simulink model "hyd_hyb_2_int_cont_1_new3_5cyc"  (Simulink 7.7)
settings: Solver=ode4  StartTime=0  StopTime=600  FixedStep=0.01  MaxStep=.4  RelTol=1e-8
InitFcn:
  | const_3_new
values from neighbouring files (symbols this model uses):
  Af = 6.3    [brk_enrg.m]    (also in brk_enrg_1.m)
  BSFC_906_205 = [3.5684 4.3466 5.1668 6.0769 6.988 7.8992 8.8104 9.7336 10.8548 11.9809 13.1112 14.2571 ... (663 elements, 39x17)]    [BSFC_map_906_205.mat]
  Cd = 0.55    [brk_enrg.m]    (also in brk_enrg_1.m)
  den = 1.202    [brk_enrg.m]    (also in brk_enrg_1.m)
  fr = 0.01    [brk_enrg.m]    (also in brk_enrg_1.m)
undefined symbols (not defined in the file or in its folder): clutchRise, down_spd_2, down_spd_3, down_spd_4, down_spd_c, Driving_Cycle, fuel_density, gearshift, I_wheel, Idle_spd_eng_906, M1_hydr, p_v_15, pmax_125, qv_125, Rw, sec_eng_sfc_map, T0, T1, T_max_906, T_min, TC_spd_ratio_max, teta_new_10, torque_convertor, Twait, up_spd_1, up_spd_2, up_spd_3, up_spd_c, v_p_10, v_p_15, v_p_5
libraries: neural x1742, fl_lib x43, nesl_utility x30, sdl_lib x14, simulink x5, simulink_need_slupdate x1
(part 47 of 47)

== matlab function controller1/online_fuz_clust/online_fuz_clus_main/new_feature_gen1/MATLAB Function ==
  data: t input double; prc_spd_0_10 output double; Driving_Cycle parameter double; T0 parameter double; T1 parameter double; prc_spd_20_30 output double; max_acc output double; prc_acc_02_04 output double; prc_acc_06_08 output double; prc_dec_02_04 output double; prc_dec_06_08 output double; rpa output double; prc_av_0_5 output double; pke output double; mem input double
function [prc_spd_0_10, prc_spd_20_30, max_acc, prc_acc_02_04,...
     prc_acc_06_08, prc_dec_02_04, prc_dec_06_08, rpa, prc_av_0_5, pke] = fcn(t, Driving_Cycle, T0, T1,mem)
 %------------------------------------------------------------------------------
cyc=Driving_Cycle;
h=size(cyc);
cyc_tim=h(1,1)+1;
%-------------------------------------------------------------------------------
sum_spd=0;a=zeros(cyc_tim,2);sum_acc=0;sum_dec=0;dis=zeros(cyc_tim,1);dist=0;
num_acc=0;num_dec=0;av=zeros(cyc_tim,2);sum_av=0;sum_av_p=0;pke_1=0;pke_dis=0;
% num_stop=0;
num_spd_0_10=0;
% num_spd_10_20=0;
num_spd_20_30=0;
% num_spd_30_40=0;num_spd_40_50=0;num_spd_50_up=0;
% num_acc_0_02=0;
num_acc_02_04=0;
% num_acc_04_06=0;
num_acc_06_08=0;
% num_acc_08_1=0;num_acc_1_up=0;
% num_dec_0_02=0;
num_dec_02_04=0;
% num_dec_04_06=0;
num_dec_06_08=0;
% num_dec_08_1=0;num_dec_1_dn=0;
num_av_0_5=0;
% num_av_5_0=0;num_av_5_10=0;num_av_10_5=0;num_av_10_up=0;num_av_10_dn=0;
%---------------------------------------------------------------------------------    
prc_spd_0_10=0;
prc_spd_20_30=0;
max_acc=0;
prc_acc_02_04=0;
prc_acc_06_08=0;
prc_dec_02_04=0;
prc_dec_06_08=0;
rpa=0;
prc_av_0_5=0;
pke=0;
%-----------------------------------------------------------------------------
if t>=T0
    r=rem(t,T1);
    if r==0                    
            for i=1:t
                sum_spd=cyc(round(i),2)+sum_spd;
                if round(i)>1
                    a(i,1)=cyc(round(i),1);
                    a(i,2)=(cyc(round(i),2)-cyc(round(i)-1,2))*(10/36)/1;
                end
                if a(round(i),2)>0
                    num_acc=num_acc+1;
                    sum_acc=sum_acc+a(round(i),2);
                    sum_av_p=sum_av_p+(0.5*((a(round(i),2)*cyc(round(i),2)*(10/36))+(a(round(i)+1,2)*cyc(round(i)+1,2)*(10/36))));% trapezoidal formula for integral
                end
                if a(round(i),2)<0
                    num_dec=num_dec+1;
                    sum_dec=sum_dec+a(round(i),2);
                end
                av(round(i),1)=cyc(round(i),1);
                av(round(i),2)=a(i,2)*(cyc(round(i),2)*10/36);
                sum_av=sum_av+av(round(i),2);
            end
            %------------------------------------------------------------------------
            % speed
            % avg_spd=sum_spd/cyc_tim;
            % max_spd=max(cyc(:,2));
            for i=1:t
                if cyc(round(i),2)<10
                    num_spd_0_10=num_spd_0_10+1;
                end
            %     if cyc(i,2)<20 && cyc(i,2)>=10
            %         num_spd_10_20=num_spd_10_20+1;
            %     end
                if cyc(round(i),2)<30 && cyc(round(i),2)>=20
                    num_spd_20_30=num_spd_20_30+1;
                end
            %     if cyc(i,2)<40 && cyc(i,2)>=30
            %         num_spd_30_40=num_spd_30_40+1;
            %     end
            %     if cyc(i,2)<50 && cyc(i,2)>=40
            %         num_spd_40_50=num_spd_40_50+1;
            %     end
            %     if cyc(i,2)>=50
            %         num_spd_50_up=num_spd_50_up+1;
            %     end
            end
            prc_spd_0_10=1*num_spd_0_10/t;
            % prc_spd_10_20=100*num_spd_10_20/cyc_tim;
            prc_spd_20_30=1*num_spd_20_30/t;
            % prc_spd_30_40=100*num_spd_30_40/cyc_tim;
            % prc_spd_40_50=100*num_spd_40_50/cyc_tim;
            % prc_spd_50_up=100*num_spd_50_up/cyc_tim;
            % out_spd=[avg_spd max_spd prc_spd_0_10 prc_spd_10_20 prc_spd_20_30 prc_spd_30_40 prc_spd_40_50 prc_spd_50_up];
            %------------------------------------------------------------------------
            %distance
            for i=1:t
                dis(round(i))=1*cyc(round(i),2)*10/36;% m
                dist=dist+dis(round(i));
            end
            %------------------------------------------------------------------------
            % acceleration
            % avg_acc=sum_acc/num_acc;
            max_acc=max(a(:,2))/6;
            for i=1:t
            %     if a(i,2)<0.2 && a(i,2)>0
            %         num_acc_0_02=num_acc_0_02+1;
            %     end
                if a(round(i),2)<0.4 && a(round(i),2)>=0.2
                    num_acc_02_04=num_acc_02_04+1;
                end
            %     if a(i,2)<0.6 && a(i,2)>=0.4
            %         num_acc_04_06=num_acc_04_06+1;
            %     end
                if a(round(i),2)<0.8 && a(round(i),2)>=0.6
                    num_acc_06_08=num_acc_06_08+1;
                end
            %     if a(i,2)<1 && a(i,2)>=0.8
            %         num_acc_08_1=num_acc_08_1+1;
            %     end
            %     if a(i,2)>=1
            %         num_acc_1_up=num_acc_1_up+1;
            %     end
            end
            % prc_acc_0_02=100*num_acc_0_02/num_acc;
            prc_acc_02_04=1*num_acc_02_04/num_acc;
            % prc_acc_04_06=100*num_acc_04_06/num_acc;
            prc_acc_06_08=1*num_acc_06_08/num_acc;
            % prc_acc_08_1=100*num_acc_08_1/num_acc;
            % prc_acc_1_up=100*num_acc_1_up/num_acc;
            % out_acc=[avg_acc max_acc prc_acc_0_02 prc_acc_02_04 prc_acc_04_06 prc_acc_06_08 prc_acc_08_1 prc_acc_1_up];
            % -----------------------------------------------------------------------
            % deceleration
            % avg_dec=sum_dec/num_dec;
            % max_dec=min(a(:,2));
            for i=1:t
            %     if a(i,2)<0 && a(i,2)>=-0.2
            %         num_dec_0_02=num_dec_0_02+1;
            %     end
                if a(round(i),2)<-0.2 && a(round(i),2)>=-0.4
                    num_dec_02_04=num_dec_02_04+1;
                end
            %     if a(i,2)<-0.4 && a(i,2)>=-0.6
            %         num_dec_04_06=num_dec_04_06+1;
            %     end
                if a(round(i),2)<-0.6 && a(round(i),2)>=-0.8
                    num_dec_06_08=num_dec_06_08+1;
                end
            %     if a(i,2)<-0.8 && a(i,2)>=-1
            %         num_dec_08_1=num_dec_08_1+1;
            %     end
            %     if a(i,2)<-1
            %         num_dec_1_dn=num_dec_1_dn+1;
            %     end
            end
            % prc_dec_0_02=100*num_dec_0_02/num_dec;
            prc_dec_02_04=1*num_dec_02_04/num_dec;
            % prc_dec_04_06=100*num_dec_04_06/num_dec;
            prc_dec_06_08=1*num_dec_06_08/num_dec;
            % prc_dec_08_1=100*num_dec_08_1/num_dec;
            % prc_dec_1_dn=100*num_dec_1_dn/num_dec;
            % out_dec=[avg_dec max_dec prc_dec_0_02 prc_dec_02_04 prc_dec_04_06 prc_dec_06_08 prc_dec_08_1 prc_dec_1_dn];
            %------------------------------------------------------------------------
            % av
            % avg_av=sum_av/cyc_tim;
            % max_av=max(av(:,2));
            % min_av=min(av(:,2));
            for i=1:t
                if av(round(i),2)<5 && av(round(i),2)>=0
                    num_av_0_5=num_av_0_5+1;
                end
            %     if av(i,2)<0 && av(i,2)>=-5
            %         num_av_5_0=num_av_5_0+1;
            %     end
            %     if av(i,2)<10 && av(i,2)>=5
            %         num_av_5_10=num_av_5_10+1;
            %     end
            %     if av(i,2)<-5 && av(i,2)>=-10
            %         num_av_10_5=num_av_10_5+1;
            %     end
            %     if av(i,2)>=10
            %         num_av_10_up=num_av_10_up+1;
            %     end
            %     if av(i,2)<-10
            %         num_av_10_dn=num_av_10_dn+1;
            %     end
            end
            prc_av_0_5=1*num_av_0_5/t;
            % prc_av_5_0=100*num_av_5_0/cyc_tim;
            % prc_av_5_10=100*num_av_5_10/cyc_tim;
            % prc_av_10_5=100*num_av_10_5/cyc_tim;
            % prc_av_10_up=100*num_av_10_up/cyc_tim;
            % prc_av_10_dn=100*num_av_10_dn/cyc_tim;
            %relative positive acceleration
            rpa=(sum_av_p/dist)/0.2;
            % out_av=[rpa prc_av_0_5 prc_av_5_0 prc_av_5_10 prc_av_10_5 prc_av_10_up prc_av_10_dn 0];
            % -----------------------------------------------------------------------
            % number of stops per km & Positive Kinetic Energy (pke)
            for i=2:t
            %     if cyc(i,2)==0
            %         if cyc(i-1,2)>0
            %             num_stop=num_stop+1;
            %         end
            %     end
                if a(round(i),2)>0
                    pke_1=pke_1+(((cyc(round(i)+1,2)*(10/36))^2)-((cyc(round(i),2)*(10/36))^2));
                    pke_dis=pke_dis+(cyc(round(i),2)*(10/36)*1); 
                end
            end
            % num_stop_km=num_stop/(dist/1000);
            pke=(pke_1/pke_dis)/2;
            % out_stop=[num_stop num_stop_km pke dist 0 0 0 0];
            %------------------------------------------------------------------------
            % outputs
            % out=[out_spd;out_acc;out_dec;out_av;out_stop];
            % sum_av_p
            % av
    else
        prc_spd_0_10=mem(1);
        prc_spd_20_30=mem(2);
        max_acc=mem(3);
        prc_acc_02_04=mem(4);
        prc_acc_06_08=mem(5);
        prc_dec_02_04=mem(6);
        prc_dec_06_08=mem(7);
        rpa=mem(8);
        prc_av_0_5=mem(9);
        pke=mem(10);
    end        
end
end

== stateflow chart hydraulic transmission/PM_hydraulic_machine /qv - pmax evaluation/Chart ==
  state state1
    during: u1=1;
  state state2
    during: u1=0;
  (default) -> state1
  state1 -> state2 : [soc <= soc_min]
  state2 -> state1 : [soc > 50]
  data: soc input double; soc_min input double; u1 output double

== matlab function hydraulic transmission/Sensor(PM)/MATLAB Function - pump ==
  data: T input double; y output double; w input double
function y = fcn(T,w)
if T<0
    y=-T*w/1000;
else
    y=0;
end
end

== matlab function hydraulic transmission/Sensor(PM)/MATLAB Function - motor ==
  data: T input double; y output double; w input double
function y = fcn(T,w)
if T>0
    y=T*w/1000;
else
    y=0;
end
end

== matlab function Engine/Sensor(eng)/MATLAB Function - ICE ==
  data: T input double; y output double; w input double
function y = fcn(T,w)
if T>0
    y=T*w/1000;
else
    y=0;
end
end

== matlab function Vehicle Dynamic/Sensor(follower)/MATLAB Function - wheel ==
  data: T input double; y output double; w input double
function y = fcn(T,w)
if T>0
    y=T*w/1000;
else
    y=0;
end
end

== matlab function Engine/eng_torq_estimator/MATLAB Function - ICE ==
  data: T input double; y output double; w input double
function y = fcn(T,w)
if T>0
    y=T*w/1000;
else
    y=0;
end
end

== matlab function controller1/online_fuz_clust/online_fuz_clus_main/Fuzz weights ==
  data: x_feat input double; u output double; teta_new_10 parameter double; init input double; t input double; T0 parameter double; T1 parameter double; dist output double; mem input double
 function [u, dist] = fcn(x_feat,teta_new_10,init,t, T0, T1,mem)
q=2;dist=zeros(4,1);
u1=zeros(4,1);
u=init;
if t>=T0
    r=rem(t,T1);
%     r1=rem(t,T0);
    if r <= 0.01         
        for i=1:4
            dist(i)=(x_feat-teta_new_10(:,i))'*(x_feat-teta_new_10(:,i));
        end                
        for k=1:4
            den=0;
            for t=1:4
                den=den+((dist(k)/dist(t))^(1/(q-1)));
            end
            u1(k)=1/(den);
        end        
        u=u1;
    else
        u=mem;
    end
end
end
